simulink model "ClimaEgyMgr"  (R2022b)
settings: Solver=FixedStepDiscrete  StartTime=0.0  StopTime=10.0  FixedStep=0.2  RelTol=1e-3
(part 2 of 2)

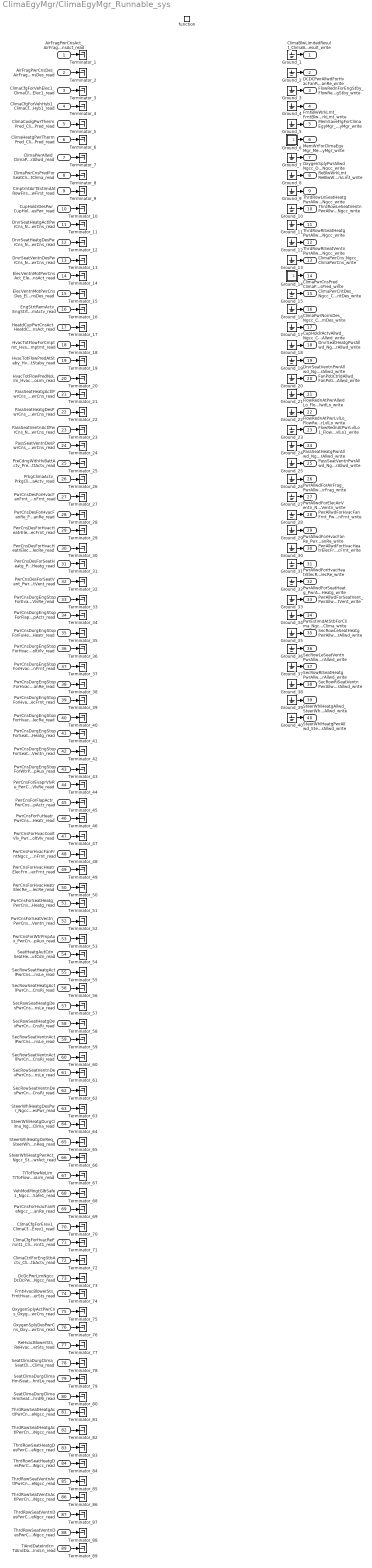
== system ClimaEgyMgr/ClimaEgyMgr_Runnable_sys ==
  AirFragPwrCnsAct_AirFragPwrCnsAct_read : Inport
  AirFragPwrCnsDes_AirFragPwrCnsDes_read : Inport  Port=2
  ClimaCfgForVehElec1_ClimaCfgForVehElec1_read : Inport  Port=3
  ClimaCfgForVehHyb1_ClimaCfgForVehHyb1_read : Inport  Port=4
  ClimaCoolgPwrThermPred_ClimaCoolgPwrThermPred_read : Inport  Port=5
  ClimaHeatgPwrThermPred_ClimaHeatgPwrThermPred_read : Inport  Port=6
  ClimaPwrAllwd_ClimaPwrAllwd_read : Inport  Port=7
  ClimaPwrCnsPredForSeatClima_ClimaPwrCnsPredForSeatClima_read : Inport  Port=8
  CmptmtAirTEstimdAtRowFirst_CmptmtAirTEstimdAtRowFirst_read : Inport  Port=9
  CupHoldrDesPwr_CupHoldrDesPwr_read : Inport  Port=10
  DrvrSeatHeatgActlPwrCns_Ngcc_DrvrSeatHeatgActlPwrCns_read : Inport  Port=11
  DrvrSeatHeatgDesPwrCns_Ngcc_DrvrSeatHeatgDesPwrCns_read : Inport  Port=12
  DrvrSeatVentnDesPwrCns_Ngcc_DrvrSeatVentnDesPwrCns_read : Inport  Port=13
  ElecVentnMotPwrCnsAct_ElecVentnMotPwrCnsAct_read : Inport  Port=14
  ElecVentnMotPwrCnsDes_ElecVentnMotPwrCnsDes_read : Inport  Port=15
  EngStrtRemActv_EngStrtRemActv_read : Inport  Port=16
  HeatdCupPwrCnsAct_HeatdCupPwrCnsAct_read : Inport  Port=17
  HvacTotFlowForCmptmt_HvacTotFlowForCmptmt_read : Inport  Port=18
  HvacTotFlowPredAtStaby_HvacTotFlowPredAtStaby_read : Inport  Port=19
  HvacTotFlowPredNoLim_HvacTotFlowPredNoLim_read : Inport  Port=20
  PassSeatHeatgActlPwrCns_Ngcc_PassSeatHeatgActlPwrCns_read : Inport  Port=21
  PassSeatHeatgDesPwrCns_Ngcc_PassSeatHeatgDesPwrCns_read : Inport  Port=22
  PassSeatVentnActlPwrCns_Ngcc_PassSeatVentnActlPwrCns_read : Inport  Port=23
  PassSeatVentnDesPwrCns_Ngcc_PassSeatVentnDesPwrCns_read : Inport  Port=24
  PreCdngWithHvBattActv_PreCdngWithHvBattActv_read : Inport  Port=25
  PrkgClimaActv_PrkgClimaActv_read : Inport  Port=26
  PwrCnsDesForHvacFanFrnt_PwrCnsDesForHvacFanFrnt_read : Inport  Port=27
  PwrCnsDesForHvacFanRe_PwrCnsDesForHvacFanRe_read : Inport  Port=28
  PwrCnsDesForHvacHeatrElecFrnt_PwrCnsDesForHvacHeatrElecFrnt_read : Inport  Port=29
  PwrCnsDesForHvacHeatrElecRe_PwrCnsDesForHvacHeatrElecRe_read : Inport  Port=30
  PwrCnsDesForSeatHeatg_PwrCnsDesForSeatHeatg_read : Inport  Port=31
  PwrCnsDesForSeatVent_PwrCnsDesForSeatVent_read : Inport  Port=32
  PwrCnsDurgEngStopForEvaprVlvRe_PwrCnsDurgEngStopForEvaprVlvRe_read : Inport  Port=33
  PwrCnsDurgEngStopForFlapActr_PwrCnsDurgEngStopForFlapActr_read : Inport  Port=34
  PwrCnsDurgEngStopForFuHeatr_PwrCnsDurgEngStopForFuHeatr_read : Inport  Port=35
  PwrCnsDurgEngStopForHvacCooltVlv_PwrCnsDurgEngStopForHvacCooltVlv_read : Inport  Port=36
  PwrCnsDurgEngStopForHvacFanFrnt_PwrCnsDurgEngStopForHvacFanFrnt_read : Inport  Port=37
  PwrCnsDurgEngStopForHvacFanRe_PwrCnsDurgEngStopForHvacFanRe_read : Inport  Port=38
  PwrCnsDurgEngStopForHvacHeatrElecFrnt_PwrCnsDurgEngStopForHvacHeatrElecFrnt_read : Inport  Port=39
  PwrCnsDurgEngStopForHvacHeatrElecRe_PwrCnsDurgEngStopForHvacHeatrElecRe_read : Inport  Port=40
  PwrCnsDurgEngStopForSeatHeatg_PwrCnsDurgEngStopForSeatHeatg_read : Inport  Port=41
  PwrCnsDurgEngStopForSeatVentn_PwrCnsDurgEngStopForSeatVentn_read : Inport  Port=42
  PwrCnsDurgEngStopForWtrPmpAux_PwrCnsDurgEngStopForWtrPmpAux_read : Inport  Port=43
  PwrCnsForEvaprVlvRe_PwrCnsForEvaprVlvRe_read : Inport  Port=44
  PwrCnsForFlapActr_PwrCnsForFlapActr_read : Inport  Port=45
  PwrCnsForFuHeatr_PwrCnsForFuHeatr_read : Inport  Port=46
  PwrCnsForHvacCooltVlv_PwrCnsForHvacCooltVlv_read : Inport  Port=47
  PwrCnsForHvacFanFrntNgcc_PwrCnsForHvacFanFrnt_read : Inport  Port=48
  PwrCnsForHvacHeatrElecFrnt_PwrCnsForHvacHeatrElecFrnt_read : Inport  Port=49
  PwrCnsForHvacHeatrElecRe_PwrCnsForHvacHeatrElecRe_read : Inport  Port=50
  PwrCnsForSeatHeatg_PwrCnsForSeatHeatg_read : Inport  Port=51
  PwrCnsForSeatVentn_PwrCnsForSeatVentn_read : Inport  Port=52
  PwrCnsForWtrPmpAux_PwrCnsForWtrPmpAux_read : Inport  Port=53
  SeatHeatgAutCdn_SeatHeatgAutCdn_read : Inport  Port=54
  SecRowSeatHeatgActlPwrCnsLe_Ngcc_SecRowSeatHeatgActlPwrCnsLe_read : Inport  Port=55
  SecRowSeatHeatgActlPwrCnsRi_Ngcc_SecRowSeatHeatgActlPwrCnsRi_read : Inport  Port=56
  SecRowSeatHeatgDesPwrCnsLe_Ngcc_SecRowSeatHeatgDesPwrCnsLe_read : Inport  Port=57
  SecRowSeatHeatgDesPwrCnsRi_Ngcc_SecRowSeatHeatgDesPwrCnsRi_read : Inport  Port=58
  SecRowSeatVentnActlPwrCnsLe_Ngcc_SecRowSeatVentnActlPwrCnsLe_read : Inport  Port=59
  SecRowSeatVentnActlPwrCnsRi_Ngcc_SecRowSeatVentnActlPwrCnsRi_read : Inport  Port=60
  SecRowSeatVentnDesPwrCnsLe_Ngcc_SecRowSeatVentnDesPwrCnsLe_read : Inport  Port=61
  SecRowSeatVentnDesPwrCnsRi_Ngcc_SecRowSeatVentnDesPwrCnsRi_read : Inport  Port=62
  SteerWhlHeatgDesPwr_Ngcc_SteerWhlHeatgDesPwr_read : Inport  Port=63
  SteerWhlHeatgDurgClima_Ngcc_SteerWhlHeatgDurgClima_read : Inport  Port=64
  SteerWhlHeatgOnReq_SteerWhlHeatgOnReq_read : Inport  Port=65
  SteerWhlHeatgPwrAct_Ngcc_SteerWhlHeatgPwrAct_read : Inport  Port=66
  TiToFlowNoLim_TiToFlowNoLim_read : Inport  Port=67
  VehModMngtGlbSafe1_Ngcc_VehModMngtGlbSafe1_read : Inport  Port=68
  PwrCnsForHvacFanReNgcc_PwrCnsForHvacFanRe_read : Inport  Port=69
  ClimaCfgForErev1_ClimaCfgForErev1_read : Inport  Port=70
  ClimaCfgForHvacRePrsnt1_ClimaCfgForHvacRePrsnt1_read : Inport  Port=71
  ClimaCtrlForEngStbActv_ClimaCtrlForEngStbActv_read : Inport  Port=72
  DcDcPwrLimNgcc_DcDcPwrLimNgcc_read : Inport  Port=73
  FrntHvacBlowerSts_FrntHvacBlowerSts_read : Inport  Port=74
  OxygenSplyActPwrCns_OxygenSplyActPwrCns_read : Inport  Port=75
  OxygenSplyDesPwrCns_OxygenSplyDesPwrCns_read : Inport  Port=76
  ReHvacBlowerSts_ReHvacBlowerSts_read : Inport  Port=77
  SeatClimaDurgClima_SeatClimaDurgClima_read : Inport  Port=78
  SeatClimaDurgClimaHmiSeatHeatgForRowThrdLe_SeatClimaDurgClimaHmiSeatHeatgForRowThrdLe_read : Inport  Port=79
  SeatClimaDurgClimaHmiSeatHeatgForRowThrdRi_SeatClimaDurgClimaHmiSeatHeatgForRowThrdRi_read : Inport  Port=80
  ThrdRowSeatHeatgActlPwrCnsLeNgcc_ThrdRowSeatHeatgActlPwrCnsLeNgcc_read : Inport  Port=81
  ThrdRowSeatHeatgActlPwrCnsRiNgcc_ThrdRowSeatHeatgActlPwrCnsRiNgcc_read : Inport  Port=82
  ThrdRowSeatHeatgDesPwrCnsLeNgcc_ThrdRowSeatHeatgDesPwrCnsLeNgcc_read : Inport  Port=83
  ThrdRowSeatHeatgDesPwrCnsRiNgcc_ThrdRowSeatHeatgDesPwrCnsRiNgcc_read : Inport  Port=84
  ThrdRowSeatVentnActlPwrCnsLeNgcc_ThrdRowSeatVentnActlPwrCnsLeNgcc_read : Inport  Port=85
  ThrdRowSeatVentnActlPwrCnsRiNgcc_ThrdRowSeatVentnActlPwrCnsRiNgcc_read : Inport  Port=86
  ThrdRowSeatVentnDesPwrCnsLeNgcc_ThrdRowSeatVentnDesPwrCnsLeNgcc_read : Inport  Port=87
  ThrdRowSeatVentnDesPwrCnsRiNgcc_ThrdRowSeatVentnDesPwrCnsRiNgcc_read : Inport  Port=88
  TiAndDateIndcn_TiAndDateIndcn_read : Inport  Port=89
  function : TriggerPort  TriggerType=function-call  VariantControl=(inherit)  SampleTimeType=periodic  SampleTime=-1
  Ground_{1..5,7..13,15..40} (38 alike) : Ground
  Ground_14 : Subsystem
  Ground_6 : Subsystem
  Terminator_{1..89} (89 alike) : Terminator
  ClimaBlwLimitedResult_ClimaBlwLimitedResult_write : Outport
      settings: EnsureOutportIsVirtual=on
  DCDCPwrAllwdForHvacFanRe_DCDCPwrAllwdForHvacFanRe_write : Outport  Port=2
      settings: EnsureOutportIsVirtual=on
  FlowRednForEngStby_FlowRednForEngStby_write : Outport  Port=3
      settings: EnsureOutportIsVirtual=on
  FrntBlwWrkLmt_FrntBlwWrkLmt_write : Outport  Port=4
      settings: EnsureOutportIsVirtual=on
  MemSaveFlgForClimaEgyMgr_MemSaveFlgForClimaEgyMgr_write : Outport  Port=5
      settings: EnsureOutportIsVirtual=on
  MemWrForClimaEgyMgr_MemWrForClimaEgyMgr_write : Outport  Port=6
      settings: EnsureOutportIsVirtual=on
  OxygenSplyPwrAllwdNgcc_OxygenSplyPwrAllwdNgcc_write : Outport  Port=7
      settings: EnsureOutportIsVirtual=on
  ReBlwWrkLmt_ReBlwWrkLmt_write : Outport  Port=8
      settings: EnsureOutportIsVirtual=on
  ThrdRowLeSeatHeatgPwrAllwdNgcc_ThrdRowLeSeatHeatgPwrAllwdNgcc_write : Outport  Port=9
      settings: EnsureOutportIsVirtual=on
  ThrdRowLeSeatVentnPwrAllwdNgcc_ThrdRowLeSeatVentnPwrAllwdNgcc_write : Outport  Port=10
      settings: EnsureOutportIsVirtual=on
  ThrdRowRiSeatHeatgPwrAllwdNgcc_ThrdRowRiSeatHeatgPwrAllwdNgcc_write : Outport  Port=11
      settings: EnsureOutportIsVirtual=on
  ThrdRowRiSeatVentnPwrAllwdNgcc_ThrdRowRiSeatVentnPwrAllwdNgcc_write : Outport  Port=12
      settings: EnsureOutportIsVirtual=on
  ClimaPwrCns_Ngcc_ClimaPwrCns_write : Outport  Port=13
      settings: EnsureOutportIsVirtual=on
  ClimaPwrCnsPred_ClimaPwrCnsPred_write : Outport  Port=14
      settings: EnsureOutportIsVirtual=on
  ClimaPwrCritDes_Ngcc_ClimaPwrCritDes_write : Outport  Port=15
      settings: EnsureOutportIsVirtual=on
  ClimaPwrNormDes_Ngcc_ClimaPwrNormDes_write : Outport  Port=16
      settings: EnsureOutportIsVirtual=on
  CupHoldrActvAllwd_Ngcc_CupHoldrActvAllwd_write : Outport  Port=17
      settings: EnsureOutportIsVirtual=on
  DrvrSeatHeatgPwrAllwd_Ngcc_DrvrSeatHeatgPwrAllwd_write : Outport  Port=18
      settings: EnsureOutportIsVirtual=on
  DrvrSeatVentnPwrAllwd_Ngcc_DrvrSeatVentnPwrAllwd_write : Outport  Port=19
      settings: EnsureOutportIsVirtual=on
  FanPotiCtrldAllwd_FanPotiCtrldAllwd_write : Outport  Port=20
      settings: EnsureOutportIsVirtual=on
  FlowRednAtPwrAllwdLo_FlowRednAtPwrAllwdLo_write : Outport  Port=21
      settings: EnsureOutportIsVirtual=on
  FlowRednAtPwrLvlLo_FlowRednAtPwrLvlLo_write : Outport  Port=22
      settings: EnsureOutportIsVirtual=on
  FlowRednAtPwrLvlLo1_FlowRednAtPwrLvlLo1_write : Outport  Port=23
      settings: EnsureOutportIsVirtual=on
  PassSeatHeatgPwrAllwd_Ngcc_PassSeatHeatgPwrAllwd_write : Outport  Port=24
      settings: EnsureOutportIsVirtual=on
  PassSeatVentnPwrAllwd_Ngcc_PassSeatVentnPwrAllwd_write : Outport  Port=25
      settings: EnsureOutportIsVirtual=on
  PwrAllwdForAirFrag_PwrAllwdForAirFrag_write : Outport  Port=26
      settings: EnsureOutportIsVirtual=on
  PwrAllwdForElecAirVentn_Ngcc_PwrAllwdForElecAirVentn_write : Outport  Port=27
      settings: EnsureOutportIsVirtual=on
  PwrAllwdForHvacFanFrnt_PwrAllwdForHvacFanFrnt_write : Outport  Port=28
      settings: EnsureOutportIsVirtual=on
  PwrAllwdForHvacFanRe_PwrAllwdForHvacFanRe_write : Outport  Port=29
      settings: EnsureOutportIsVirtual=on
  PwrAllwdForHvacHeatrElecFrnt_PwrAllwdForHvacHeatrElecFrnt_write : Outport  Port=30
      settings: EnsureOutportIsVirtual=on
  PwrAllwdForHvacHeatrElecRe_PwrAllwdForHvacHeatrElecRe_write : Outport  Port=31
      settings: EnsureOutportIsVirtual=on
  PwrAllwdForSeatHeatg_PwrAllwdForSeatHeatg_write : Outport  Port=32
      settings: EnsureOutportIsVirtual=on
  PwrAllwdForSeatVent_PwrAllwdForSeatVent_write : Outport  Port=33
      settings: EnsureOutportIsVirtual=on
  PwrEstimdAtStbForClima_Ngcc_PwrEstimdAtStbForClima_write : Outport  Port=34
      settings: EnsureOutportIsVirtual=on
  SecRowLeSeatHeatgPwrAllwd_Ngcc_SecRowLeSeatHeatgPwrAllwd_write : Outport  Port=35
      settings: EnsureOutportIsVirtual=on
  SecRowLeSeatVentnPwrAllwd_Ngcc_SecRowLeSeatVentnPwrAllwd_write : Outport  Port=36
      settings: EnsureOutportIsVirtual=on
  SecRowRiSeatHeatgPwrAllwd_Ngcc_SecRowRiSeatHeatgPwrAllwd_write : Outport  Port=37
      settings: EnsureOutportIsVirtual=on
  SecRowRiSeatVentnPwrAllwd_Ngcc_SecRowRiSeatVentnPwrAllwd_write : Outport  Port=38
      settings: EnsureOutportIsVirtual=on
  SteerWhlHeatgAllwd_SteerWhlHeatgAllwd_write : Outport  Port=39
      settings: EnsureOutportIsVirtual=on
  SteerWhlHeatgPwrAllwd_SteerWhlHeatgPwrAllwd_write : Outport  Port=40
      settings: EnsureOutportIsVirtual=on
  wires:
  AirFragPwrCnsAct_AirFragPwrCnsAct_read/1 -> Terminator_1/1
  AirFragPwrCnsDes_AirFragPwrCnsDes_read/1 -> Terminator_2/1
  ClimaCfgForVehElec1_ClimaCfgForVehElec1_read/1 -> Terminator_3/1
  ClimaCfgForVehHyb1_ClimaCfgForVehHyb1_read/1 -> Terminator_4/1
  ClimaCoolgPwrThermPred_ClimaCoolgPwrThermPred_read/1 -> Terminator_5/1
  ClimaHeatgPwrThermPred_ClimaHeatgPwrThermPred_read/1 -> Terminator_6/1
  ClimaPwrAllwd_ClimaPwrAllwd_read/1 -> Terminator_7/1
  ClimaPwrCnsPredForSeatClima_ClimaPwrCnsPredForSeatClima_read/1 -> Terminator_8/1
  CmptmtAirTEstimdAtRowFirst_CmptmtAirTEstimdAtRowFirst_read/1 -> Terminator_9/1
  CupHoldrDesPwr_CupHoldrDesPwr_read/1 -> Terminator_10/1
  DrvrSeatHeatgActlPwrCns_Ngcc_DrvrSeatHeatgActlPwrCns_read/1 -> Terminator_11/1
  DrvrSeatHeatgDesPwrCns_Ngcc_DrvrSeatHeatgDesPwrCns_read/1 -> Terminator_12/1
  DrvrSeatVentnDesPwrCns_Ngcc_DrvrSeatVentnDesPwrCns_read/1 -> Terminator_13/1
  ElecVentnMotPwrCnsAct_ElecVentnMotPwrCnsAct_read/1 -> Terminator_14/1
  ElecVentnMotPwrCnsDes_ElecVentnMotPwrCnsDes_read/1 -> Terminator_15/1
  EngStrtRemActv_EngStrtRemActv_read/1 -> Terminator_16/1
  HeatdCupPwrCnsAct_HeatdCupPwrCnsAct_read/1 -> Terminator_17/1
  HvacTotFlowForCmptmt_HvacTotFlowForCmptmt_read/1 -> Terminator_18/1
  HvacTotFlowPredAtStaby_HvacTotFlowPredAtStaby_read/1 -> Terminator_19/1
  HvacTotFlowPredNoLim_HvacTotFlowPredNoLim_read/1 -> Terminator_20/1
  PassSeatHeatgActlPwrCns_Ngcc_PassSeatHeatgActlPwrCns_read/1 -> Terminator_21/1
  PassSeatHeatgDesPwrCns_Ngcc_PassSeatHeatgDesPwrCns_read/1 -> Terminator_22/1
  PassSeatVentnActlPwrCns_Ngcc_PassSeatVentnActlPwrCns_read/1 -> Terminator_23/1
  PassSeatVentnDesPwrCns_Ngcc_PassSeatVentnDesPwrCns_read/1 -> Terminator_24/1
  PreCdngWithHvBattActv_PreCdngWithHvBattActv_read/1 -> Terminator_25/1
  PrkgClimaActv_PrkgClimaActv_read/1 -> Terminator_26/1
  PwrCnsDesForHvacFanFrnt_PwrCnsDesForHvacFanFrnt_read/1 -> Terminator_27/1
  PwrCnsDesForHvacFanRe_PwrCnsDesForHvacFanRe_read/1 -> Terminator_28/1
  PwrCnsDesForHvacHeatrElecFrnt_PwrCnsDesForHvacHeatrElecFrnt_read/1 -> Terminator_29/1
  PwrCnsDesForHvacHeatrElecRe_PwrCnsDesForHvacHeatrElecRe_read/1 -> Terminator_30/1
  PwrCnsDesForSeatHeatg_PwrCnsDesForSeatHeatg_read/1 -> Terminator_31/1
  PwrCnsDesForSeatVent_PwrCnsDesForSeatVent_read/1 -> Terminator_32/1
  PwrCnsDurgEngStopForEvaprVlvRe_PwrCnsDurgEngStopForEvaprVlvRe_read/1 -> Terminator_33/1
  PwrCnsDurgEngStopForFlapActr_PwrCnsDurgEngStopForFlapActr_read/1 -> Terminator_34/1
  PwrCnsDurgEngStopForFuHeatr_PwrCnsDurgEngStopForFuHeatr_read/1 -> Terminator_35/1
  PwrCnsDurgEngStopForHvacCooltVlv_PwrCnsDurgEngStopForHvacCooltVlv_read/1 -> Terminator_36/1
  PwrCnsDurgEngStopForHvacFanFrnt_PwrCnsDurgEngStopForHvacFanFrnt_read/1 -> Terminator_37/1
  PwrCnsDurgEngStopForHvacFanRe_PwrCnsDurgEngStopForHvacFanRe_read/1 -> Terminator_38/1
  PwrCnsDurgEngStopForHvacHeatrElecFrnt_PwrCnsDurgEngStopForHvacHeatrElecFrnt_read/1 -> Terminator_39/1
  PwrCnsDurgEngStopForHvacHeatrElecRe_PwrCnsDurgEngStopForHvacHeatrElecRe_read/1 -> Terminator_40/1
  PwrCnsDurgEngStopForSeatHeatg_PwrCnsDurgEngStopForSeatHeatg_read/1 -> Terminator_41/1
  PwrCnsDurgEngStopForSeatVentn_PwrCnsDurgEngStopForSeatVentn_read/1 -> Terminator_42/1
  PwrCnsDurgEngStopForWtrPmpAux_PwrCnsDurgEngStopForWtrPmpAux_read/1 -> Terminator_43/1
  PwrCnsForEvaprVlvRe_PwrCnsForEvaprVlvRe_read/1 -> Terminator_44/1
  PwrCnsForFlapActr_PwrCnsForFlapActr_read/1 -> Terminator_45/1
  PwrCnsForFuHeatr_PwrCnsForFuHeatr_read/1 -> Terminator_46/1
  PwrCnsForHvacCooltVlv_PwrCnsForHvacCooltVlv_read/1 -> Terminator_47/1
  PwrCnsForHvacFanFrntNgcc_PwrCnsForHvacFanFrnt_read/1 -> Terminator_48/1
  PwrCnsForHvacHeatrElecFrnt_PwrCnsForHvacHeatrElecFrnt_read/1 -> Terminator_49/1
  PwrCnsForHvacHeatrElecRe_PwrCnsForHvacHeatrElecRe_read/1 -> Terminator_50/1
  PwrCnsForSeatHeatg_PwrCnsForSeatHeatg_read/1 -> Terminator_51/1
  PwrCnsForSeatVentn_PwrCnsForSeatVentn_read/1 -> Terminator_52/1
  PwrCnsForWtrPmpAux_PwrCnsForWtrPmpAux_read/1 -> Terminator_53/1
  SeatHeatgAutCdn_SeatHeatgAutCdn_read/1 -> Terminator_54/1
  SecRowSeatHeatgActlPwrCnsLe_Ngcc_SecRowSeatHeatgActlPwrCnsLe_read/1 -> Terminator_55/1
  SecRowSeatHeatgActlPwrCnsRi_Ngcc_SecRowSeatHeatgActlPwrCnsRi_read/1 -> Terminator_56/1
  SecRowSeatHeatgDesPwrCnsLe_Ngcc_SecRowSeatHeatgDesPwrCnsLe_read/1 -> Terminator_57/1
  SecRowSeatHeatgDesPwrCnsRi_Ngcc_SecRowSeatHeatgDesPwrCnsRi_read/1 -> Terminator_58/1
  SecRowSeatVentnActlPwrCnsLe_Ngcc_SecRowSeatVentnActlPwrCnsLe_read/1 -> Terminator_59/1
  SecRowSeatVentnActlPwrCnsRi_Ngcc_SecRowSeatVentnActlPwrCnsRi_read/1 -> Terminator_60/1
  SecRowSeatVentnDesPwrCnsLe_Ngcc_SecRowSeatVentnDesPwrCnsLe_read/1 -> Terminator_61/1
  SecRowSeatVentnDesPwrCnsRi_Ngcc_SecRowSeatVentnDesPwrCnsRi_read/1 -> Terminator_62/1
  SteerWhlHeatgDesPwr_Ngcc_SteerWhlHeatgDesPwr_read/1 -> Terminator_63/1
  SteerWhlHeatgDurgClima_Ngcc_SteerWhlHeatgDurgClima_read/1 -> Terminator_64/1
  SteerWhlHeatgOnReq_SteerWhlHeatgOnReq_read/1 -> Terminator_65/1
  SteerWhlHeatgPwrAct_Ngcc_SteerWhlHeatgPwrAct_read/1 -> Terminator_66/1
  TiToFlowNoLim_TiToFlowNoLim_read/1 -> Terminator_67/1
  VehModMngtGlbSafe1_Ngcc_VehModMngtGlbSafe1_read/1 -> Terminator_68/1
  PwrCnsForHvacFanReNgcc_PwrCnsForHvacFanRe_read/1 -> Terminator_69/1
  ClimaCfgForErev1_ClimaCfgForErev1_read/1 -> Terminator_70/1
  ClimaCfgForHvacRePrsnt1_ClimaCfgForHvacRePrsnt1_read/1 -> Terminator_71/1
  ClimaCtrlForEngStbActv_ClimaCtrlForEngStbActv_read/1 -> Terminator_72/1
  DcDcPwrLimNgcc_DcDcPwrLimNgcc_read/1 -> Terminator_73/1
  FrntHvacBlowerSts_FrntHvacBlowerSts_read/1 -> Terminator_74/1
  OxygenSplyActPwrCns_OxygenSplyActPwrCns_read/1 -> Terminator_75/1
  OxygenSplyDesPwrCns_OxygenSplyDesPwrCns_read/1 -> Terminator_76/1
  ReHvacBlowerSts_ReHvacBlowerSts_read/1 -> Terminator_77/1
  SeatClimaDurgClima_SeatClimaDurgClima_read/1 -> Terminator_78/1
  SeatClimaDurgClimaHmiSeatHeatgForRowThrdLe_SeatClimaDurgClimaHmiSeatHeatgForRowThrdLe_read/1 -> Terminator_79/1
  SeatClimaDurgClimaHmiSeatHeatgForRowThrdRi_SeatClimaDurgClimaHmiSeatHeatgForRowThrdRi_read/1 -> Terminator_80/1
  ThrdRowSeatHeatgActlPwrCnsLeNgcc_ThrdRowSeatHeatgActlPwrCnsLeNgcc_read/1 -> Terminator_81/1
  ThrdRowSeatHeatgActlPwrCnsRiNgcc_ThrdRowSeatHeatgActlPwrCnsRiNgcc_read/1 -> Terminator_82/1
  ThrdRowSeatHeatgDesPwrCnsLeNgcc_ThrdRowSeatHeatgDesPwrCnsLeNgcc_read/1 -> Terminator_83/1
  ThrdRowSeatHeatgDesPwrCnsRiNgcc_ThrdRowSeatHeatgDesPwrCnsRiNgcc_read/1 -> Terminator_84/1
  ThrdRowSeatVentnActlPwrCnsLeNgcc_ThrdRowSeatVentnActlPwrCnsLeNgcc_read/1 -> Terminator_85/1
  ThrdRowSeatVentnActlPwrCnsRiNgcc_ThrdRowSeatVentnActlPwrCnsRiNgcc_read/1 -> Terminator_86/1
  ThrdRowSeatVentnDesPwrCnsLeNgcc_ThrdRowSeatVentnDesPwrCnsLeNgcc_read/1 -> Terminator_87/1
  ThrdRowSeatVentnDesPwrCnsRiNgcc_ThrdRowSeatVentnDesPwrCnsRiNgcc_read/1 -> Terminator_88/1
  TiAndDateIndcn_TiAndDateIndcn_read/1 -> Terminator_89/1
  Ground_1/1 -> ClimaBlwLimitedResult_ClimaBlwLimitedResult_write/1
  Ground_2/1 -> DCDCPwrAllwdForHvacFanRe_DCDCPwrAllwdForHvacFanRe_write/1
  Ground_3/1 -> FlowRednForEngStby_FlowRednForEngStby_write/1
  Ground_4/1 -> FrntBlwWrkLmt_FrntBlwWrkLmt_write/1
  Ground_5/1 -> MemSaveFlgForClimaEgyMgr_MemSaveFlgForClimaEgyMgr_write/1
  Ground_6/1(Out1) -> MemWrForClimaEgyMgr_MemWrForClimaEgyMgr_write/1
  Ground_7/1 -> OxygenSplyPwrAllwdNgcc_OxygenSplyPwrAllwdNgcc_write/1
  Ground_8/1 -> ReBlwWrkLmt_ReBlwWrkLmt_write/1
  Ground_9/1 -> ThrdRowLeSeatHeatgPwrAllwdNgcc_ThrdRowLeSeatHeatgPwrAllwdNgcc_write/1
  Ground_10/1 -> ThrdRowLeSeatVentnPwrAllwdNgcc_ThrdRowLeSeatVentnPwrAllwdNgcc_write/1
  Ground_11/1 -> ThrdRowRiSeatHeatgPwrAllwdNgcc_ThrdRowRiSeatHeatgPwrAllwdNgcc_write/1
  Ground_12/1 -> ThrdRowRiSeatVentnPwrAllwdNgcc_ThrdRowRiSeatVentnPwrAllwdNgcc_write/1
  Ground_13/1 -> ClimaPwrCns_Ngcc_ClimaPwrCns_write/1
  Ground_14/1(Out1) -> ClimaPwrCnsPred_ClimaPwrCnsPred_write/1
  Ground_15/1 -> ClimaPwrCritDes_Ngcc_ClimaPwrCritDes_write/1
  Ground_16/1 -> ClimaPwrNormDes_Ngcc_ClimaPwrNormDes_write/1
  Ground_17/1 -> CupHoldrActvAllwd_Ngcc_CupHoldrActvAllwd_write/1
  Ground_18/1 -> DrvrSeatHeatgPwrAllwd_Ngcc_DrvrSeatHeatgPwrAllwd_write/1
  Ground_19/1 -> DrvrSeatVentnPwrAllwd_Ngcc_DrvrSeatVentnPwrAllwd_write/1
  Ground_20/1 -> FanPotiCtrldAllwd_FanPotiCtrldAllwd_write/1
  Ground_21/1 -> FlowRednAtPwrAllwdLo_FlowRednAtPwrAllwdLo_write/1
  Ground_22/1 -> FlowRednAtPwrLvlLo_FlowRednAtPwrLvlLo_write/1
  Ground_23/1 -> FlowRednAtPwrLvlLo1_FlowRednAtPwrLvlLo1_write/1
  Ground_24/1 -> PassSeatHeatgPwrAllwd_Ngcc_PassSeatHeatgPwrAllwd_write/1
  Ground_25/1 -> PassSeatVentnPwrAllwd_Ngcc_PassSeatVentnPwrAllwd_write/1
  Ground_26/1 -> PwrAllwdForAirFrag_PwrAllwdForAirFrag_write/1
  Ground_27/1 -> PwrAllwdForElecAirVentn_Ngcc_PwrAllwdForElecAirVentn_write/1
  Ground_28/1 -> PwrAllwdForHvacFanFrnt_PwrAllwdForHvacFanFrnt_write/1
  Ground_29/1 -> PwrAllwdForHvacFanRe_PwrAllwdForHvacFanRe_write/1
  Ground_30/1 -> PwrAllwdForHvacHeatrElecFrnt_PwrAllwdForHvacHeatrElecFrnt_write/1
  Ground_31/1 -> PwrAllwdForHvacHeatrElecRe_PwrAllwdForHvacHeatrElecRe_write/1
  Ground_32/1 -> PwrAllwdForSeatHeatg_PwrAllwdForSeatHeatg_write/1
  Ground_33/1 -> PwrAllwdForSeatVent_PwrAllwdForSeatVent_write/1
  Ground_34/1 -> PwrEstimdAtStbForClima_Ngcc_PwrEstimdAtStbForClima_write/1
  Ground_35/1 -> SecRowLeSeatHeatgPwrAllwd_Ngcc_SecRowLeSeatHeatgPwrAllwd_write/1
  Ground_36/1 -> SecRowLeSeatVentnPwrAllwd_Ngcc_SecRowLeSeatVentnPwrAllwd_write/1
  Ground_37/1 -> SecRowRiSeatHeatgPwrAllwd_Ngcc_SecRowRiSeatHeatgPwrAllwd_write/1
  Ground_38/1 -> SecRowRiSeatVentnPwrAllwd_Ngcc_SecRowRiSeatVentnPwrAllwd_write/1
  Ground_39/1 -> SteerWhlHeatgAllwd_SteerWhlHeatgAllwd_write/1
  Ground_40/1 -> SteerWhlHeatgPwrAllwd_SteerWhlHeatgPwrAllwd_write/1

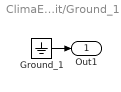
== system ClimaEgyMgr/ClimaEgyMgr_Init/Ground_1 ==
  Ground_1 : Ground
  Out1 : Outport  OutDataTypeStr=Bus: ClimaPwrCnsPredRec1  PortDimensions=1
  wires:
  Ground_1/1 -> Out1/1

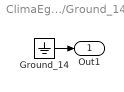
== system ClimaEgyMgr/ClimaEgyMgr_Runnable_sys/Ground_14 ==
  Ground_14 : Ground
  Out1 : Outport  OutDataTypeStr=Bus: ClimaPwrCnsPredRec1  PortDimensions=1
  wires:
  Ground_14/1 -> Out1/1

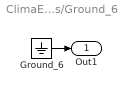
== system ClimaEgyMgr/ClimaEgyMgr_Runnable_sys/Ground_6 ==
  Ground_6 : Ground
  Out1 : Outport  OutDataTypeStr=Bus: MemRecForClimaEgyMgr  PortDimensions=1
  wires:
  Ground_6/1 -> Out1/1
